simulink model "HvacHeatrTCtrl"  (R2022b)
settings: Solver=FixedStepDiscrete  StartTime=0.0  StopTime=10.0  FixedStep=0.2  RelTol=1e-3
(part 2 of 2)

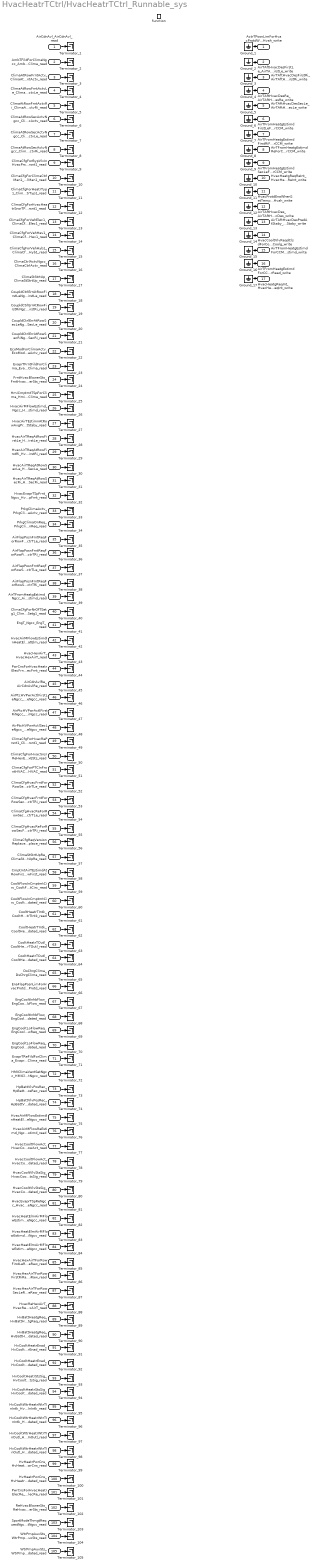
== system HvacHeatrTCtrl/HvacHeatrTCtrl_Runnable_sys ==
  AirCdnAvl_AirCdnAvl_read : Inport
  AmbTFildForClimaNgcc_AmbTFildForClima_read : Inport  Port=2
  ClimaAtRowFrntActv_ClimaAtRowFrntActv_read : Inport  Port=3
  ClimaAtRowFrntActvLe_ClimaAtRowFrntActvLe_read : Inport  Port=4
  ClimaAtRowFrntActvRi_ClimaAtRowFrntActvRi_read : Inport  Port=5
  ClimaAtRowSecActvNgcc_ClimaAtRowSecActv_read : Inport  Port=6
  ClimaAtRowSecActvNgcc_ClimaAtRowSecActvLe_read : Inport  Port=7
  ClimaAtRowSecActvNgcc_ClimaAtRowSecActvRi_read : Inport  Port=8
  ClimaCfgForBypVlvInHvacPrsnt1_ClimaCfgForBypVlvInHvacPrsnt1_read : Inport  Port=9
  ClimaCfgForClimaCtrlMan1_ClimaCfgForClimaCtrlMan1_read : Inport  Port=10
  ClimaCfgForHeatrTyp1_ClimaCfgForHeatrTyp1_read : Inport  Port=11
  ClimaCfgForHvacHeatrSnsrTPrsnt1_ClimaCfgForHvacHeatrSnsrTPrsnt1_read : Inport  Port=12
  ClimaCfgForVehElec1_ClimaCfgForVehElec1_read : Inport  Port=13
  ClimaCfgForVehHev1_ClimaCfgForVehHev1_read : Inport  Port=14
  ClimaCfgForVehHyb1_ClimaCfgForVehHyb1_read : Inport  Port=15
  ClimaCtrlActvNgcc_ClimaCtrlActv_read : Inport  Port=16
  ClimaStStrtUp_ClimaStStrtUp_read : Inport  Port=17
  CoupldCtrlErrAtRowFirstLeNgcc_CoupldCtrlErrAtRowFirstLe_read : Inport  Port=18
  CoupldCtrlErrAtRowFirstRiNgcc_CoupldCtrlErrAtRowFirstRi_read : Inport  Port=19
  CoupldCtrlErrAtRowSecLeNgcc_CoupldCtrlErrAtRowSecLe_read : Inport  Port=20
  CoupldCtrlErrAtRowSecRiNgcc_CoupldCtrlErrAtRowSecRi_read : Inport  Port=21
  EcoModForClimaActv_EcoModForClimaActv_read : Inport  Port=22
  EvaprTFrntFildForClima_EvaprTFrntFildForClima_read : Inport  Port=23
  FrntHvacBlowerSts_FrntHvacBlowerSts_read : Inport  Port=24
  HmiCmptmtTSpForClima_HmiCmptmtTSpForClima_read : Inport  Port=25
  HvacAirMFlowEstimd_Ngcc_HvacAirMFlowEstimd_read : Inport  Port=26
  HvacAirTEstimnAtRowAvgPredAtStaby_HvacAirTEstimnAtRowAvgPredAtStaby_read : Inport  Port=27
  HvacAirTReqAtRowFirstLe_HvacAirTReqAtRowFirstLe_read : Inport  Port=28
  HvacAirTReqAtRowFirstRi_HvacAirTReqAtRowFirstRi_read : Inport  Port=29
  HvacAirTReqAtRowSecLe_HvacAirTReqAtRowSecLe_read : Inport  Port=30
  HvacAirTReqAtRowSecRi_HvacAirTReqAtRowSecRi_read : Inport  Port=31
  HvacEvaprTSpFrnt_Ngcc_HvacEvaprTSpFrnt_read : Inport  Port=32
  PrkgClimaActv_PrkgClimaActv_read : Inport  Port=33
  PrkgClimaOnReq_PrkgClimaOnReq_read : Inport  Port=34
  AirFlapPosnFrntReqForRowFirstForActrTLe_AirFlapPosnFrntReqForRowFirstForActrTLe_read : Inport  Port=35
  AirFlapPosnFrntReqForRowFirstForActrTRi_AirFlapPosnFrntReqForRowFirstForActrTRi_read : Inport  Port=36
  AirFlapPosnFrntReqForRowSecForActrTLe_AirFlapPosnFrntReqForRowSecForActrTLe_read : Inport  Port=37
  AirFlapPosnFrntReqForRowSecForActrTRi_AirFlapPosnFrntReqForRowSecForActrTRi_read : Inport  Port=38
  AirTFromHeatgEstimd_Ngcc_AirTFromHeatgEstimd_read : Inport  Port=39
  ClimaCfgForNrOfTSetg1_ClimaCfgForNrOfTSetg1_read : Inport  Port=40
  EngT_Ngcc_EngT_read : Inport  Port=41
  HvacAirMFlowEstimdInHeatElm_HvacAirMFlowEstimdInHeatElm_read : Inport  Port=42
  HvacHexAirT_HvacHexAirT_read : Inport  Port=43
  PwrCnsForHvacHeatrElecFrnt_PwrCnsForHvacHeatrElecFrnt_read : Inport  Port=44
  AirCdnAvlRe_AirCdnAvlRe_read : Inport  Port=45
  AirPtcHVPwrActlFirstLeNgcc_AirPtcHVPwrActlFirstLeNgcc_read : Inport  Port=46
  AirPtcHVPwrActlFirstRiNgcc_AirPtcHVPwrActlFirstRiNgcc_read : Inport  Port=47
  AirPtcHVPwrActlSecLeNgcc_AirPtcHVPwrActlSecLeNgcc_read : Inport  Port=48
  ClimaCfgForHvacRePrsnt1_ClimaCfgForHvacRePrsnt1_read : Inport  Port=49
  ClimaCfgForHvacSnsrReHexESts_ClimaCfgForHvacSnsrReHexESts_read : Inport  Port=50
  ClimaCfgForPTCInFrontHVAC_ClimaCfgForPTCInFrontHVAC_read : Inport  Port=51
  ClimaCfgHvacFrntForRowSecForActrTLe_ClimaCfgHvacFrntForRowSecForActrTLe_read : Inport  Port=52
  ClimaCfgHvacFrntForRowSecForActrTRi_ClimaCfgHvacFrntForRowSecForActrTRi_read : Inport  Port=53
  ClimaCfgHvacReForRowSecForActrTLe_ClimaCfgHvacReForRowSecForActrTLe_read : Inport  Port=54
  ClimaCfgHvacReForRowSecForActrTRi_ClimaCfgHvacReForRowSecForActrTRi_read : Inport  Port=55
  ClimaCfgReqVersionReplace_ClimaCfgReqVersionReplace_read : Inport  Port=56
  ClimaStStrtUpRe_ClimaStStrtUpRe_read : Inport  Port=57
  CmptmtAirTEstimdAtRowFirst_CmptmtAirTEstimdAtRowFirst_read : Inport  Port=58
  CooltFlowInCmptmtCirc_CooltFlowInCmptmtCirc_read : Inport  Port=59
  CooltFlowInCmptmtCirc_CooltFlowInCmptmtCirc_IsUpdated_read : Inport  Port=60
  CooltHeatrTIntk_CooltHeatrTIntk_read : Inport  Port=61
  CooltHeatrTIntk_CooltHeatrTIntk_IsUpdated_read : Inport  Port=62
  CooltHeatrTOutl_CooltHeatrTOutl_read : Inport  Port=63
  CooltHeatrTOutl_CooltHeatrTOutl_IsUpdated_read : Inport  Port=64
  DsChrgClima_DsChrgClima_read : Inport  Port=65
  EnaFlapPosnLimForHvacProtd_EnaFlapPosnLimForHvacProtd_read : Inport  Port=66
  EngCooltInhbFlow_EngCooltInhbFlow_read : Inport  Port=67
  EngCooltInhbFlow_EngCooltInhbFlow_IsUpdated_read : Inport  Port=68
  EngCooltLoFlowReq_EngCooltLoFlowReq_read : Inport  Port=69
  EngCooltLoFlowReq_EngCooltLoFlowReq_IsUpdated_read : Inport  Port=70
  EvaprTReFildForClima_EvaprTReFildForClima_read : Inport  Port=71
  HMIClimaVentSetNgcc_HMIClimaVentSetNgcc_read : Inport  Port=72
  HpBattVlvPosRec_HpBattVlvPosRec_read : Inport  Port=73
  HpBattVlvPosRec_HpBattVlvPosRec_IsUpdated_read : Inport  Port=74
  HvacAirMFlowEstimdInHeatElmReNgcc_HvacAirMFlowEstimdInHeatElmReNgcc_read : Inport  Port=75
  HvacAirMFlowReEstimd_Ngcc_HvacAirMFlowReEstimd_read : Inport  Port=76
  HvacCooltFlowAct_HvacCooltFlowAct_read : Inport  Port=77
  HvacCooltFlowAct_HvacCooltFlowAct_IsUpdated_read : Inport  Port=78
  HvacCooltVlvStsSig_HvacCooltVlvStsSig_read : Inport  Port=79
  HvacCooltVlvStsSig_HvacCooltVlvStsSig_IsUpdated_read : Inport  Port=80
  HvacEvaprTSpReNgcc_HvacEvaprTSpReNgcc_read : Inport  Port=81
  HvacHeatElmAirMFlowEstimdFirstLeNgcc_HvacHeatElmAirMFlowEstimdFirstLeNgcc_read : Inport  Port=82
  HvacHeatElmAirMFlowEstimdFirstRiNgcc_HvacHeatElmAirMFlowEstimdFirstRiNgcc_read : Inport  Port=83
  HvacHeatElmAirMFlowEstimdSecLeNgcc_HvacHeatElmAirMFlowEstimdSecLeNgcc_read : Inport  Port=84
  HvacHexAirTForRowFirstLeRaw_HvacHexAirTForRowFirstLeRaw_read : Inport  Port=85
  HvacHexAirTForRowFirstRiRaw_HvacHexAirTForRowFirstRiRaw_read : Inport  Port=86
  HvacHexAirTForRowSecLeRaw_HvacHexAirTForRowSecLeRaw_read : Inport  Port=87
  HvacReHexAirT_HvacReHexAirT_read : Inport  Port=88
  HvBattHeatgReq_HvBattHeatgReq_read : Inport  Port=89
  HvBattHeatgReq_HvBattHeatgReq_IsUpdated_read : Inport  Port=90
  HvCooltHeatrEnad_HvCooltHeatrEnad_read : Inport  Port=91
  HvCooltHeatrEnad_HvCooltHeatrEnad_IsUpdated_read : Inport  Port=92
  HvCooltHeatrStsSig_HvCooltHeatrStsSig_read : Inport  Port=93
  HvCooltHeatrStsSig_HvCooltHeatrStsSig_IsUpdated_read : Inport  Port=94
  HvCooltWtrHeatrWtrTInIntk_HvCooltWtrHeatrWtrTInIntk_read : Inport  Port=95
  HvCooltWtrHeatrWtrTInIntk_HvCooltWtrHeatrWtrTInIntk_IsUpdated_read : Inport  Port=96
  HvCooltWtrHeatrWtrTInOutl_HvCooltWtrHeatrWtrTInOutl_read : Inport  Port=97
  HvCooltWtrHeatrWtrTInOutl_HvCooltWtrHeatrWtrTInOutl_IsUpdated_read : Inport  Port=98
  HvHeatrPwrCns_HvHeatrPwrCns_read : Inport  Port=99
  HvHeatrPwrCns_HvHeatrPwrCns_IsUpdated_read : Inport  Port=100
  PwrCnsForHvacHeatrElecRe_PwrCnsForHvacHeatrElecRe_read : Inport  Port=101
  ReHvacBlowerSts_ReHvacBlowerSts_read : Inport  Port=102
  SportModeThmgtRequestNgcc_SportModeThmgtRequestNgcc_read : Inport  Port=103
  WtrPmpAuxSts_WtrPmpAuxSts_read : Inport  Port=104
  WtrPmpAuxSts_WtrPmpAuxSts_IsUpdated_read : Inport  Port=105
  function : TriggerPort  TriggerType=function-call  VariantControl=(inherit)  SampleTimeType=periodic  SampleTime=-1
  Ground_{1..17} (17 alike) : Ground
  Terminator_{1..105} (105 alike) : Terminator
  ActrTPosnLimForHvacProtdWhenSetTempDiffWithHvah_ActrTPosnLimForHvacProtdWhenSetTempDiffWithHvah_write : Outport
      settings: EnsureOutportIsVirtual=on
  AirTAftHvacDesFirstLe_AirTAftHvacDesFirstLe_write : Outport  Port=2
      settings: EnsureOutportIsVirtual=on
  AirTAftHvacDesFirstRi_AirTAftHvacDesFirstRi_write : Outport  Port=3
      settings: EnsureOutportIsVirtual=on
  AirTAftHvacDesRe_AirTAftHvacDesRe_write : Outport  Port=4
      settings: EnsureOutportIsVirtual=on
  AirTAftHvacDesSecLe_AirTAftHvacDesSecLe_write : Outport  Port=5
      settings: EnsureOutportIsVirtual=on
  AirTFromHeatgEstimdFirstLeForCCM_AirTFromHeatgEstimdFirstLeForCCM_write : Outport  Port=6
      settings: EnsureOutportIsVirtual=on
  AirTFromHeatgEstimdFirstRiForCCM_AirTFromHeatgEstimdFirstRiForCCM_write : Outport  Port=7
      settings: EnsureOutportIsVirtual=on
  AirTFromHeatgEstimdReForCCM_AirTFromHeatgEstimdReForCCM_write : Outport  Port=8
      settings: EnsureOutportIsVirtual=on
  AirTFromHeatgEstimdSecLeForCCM_AirTFromHeatgEstimdSecLeForCCM_write : Outport  Port=9
      settings: EnsureOutportIsVirtual=on
  HvacHeatgReqReInt_HvacHeatgReqReInt_write : Outport  Port=10
      settings: EnsureOutportIsVirtual=on
  HvacProtdEnaWhenSetTempDiffWithHvah_HvacProtdEnaWhenSetTempDiffWithHvah_write : Outport  Port=11
      settings: EnsureOutportIsVirtual=on
  AirTAftHvacDes_AirTAftHvacDes_write : Outport  Port=12
      settings: EnsureOutportIsVirtual=on
  AirTAftHvacDesPredAtStaby_AirTAftHvacDesPredAtStaby_write : Outport  Port=13
      settings: EnsureOutportIsVirtual=on
  HvacCooltVlvReqdClsdForCoolg_HvacCooltVlvReqdClsdForCoolg_write : Outport  Port=14
      settings: EnsureOutportIsVirtual=on
  AirTFromHeatgEstimdForCCM_AirTFromHeatgEstimd_write : Outport  Port=15
      settings: EnsureOutportIsVirtual=on
  AirTFromHeatgEstimdForCCMForRead_AirTFromHeatgEstimdForCCMForRead_write : Outport  Port=16
      settings: EnsureOutportIsVirtual=on
  HvacHeatgReqInt_HvacHeatgReqInt_write : Outport  Port=17
      settings: EnsureOutportIsVirtual=on
  wires:
  AirCdnAvl_AirCdnAvl_read/1 -> Terminator_1/1
  AmbTFildForClimaNgcc_AmbTFildForClima_read/1 -> Terminator_2/1
  ClimaAtRowFrntActv_ClimaAtRowFrntActv_read/1 -> Terminator_3/1
  ClimaAtRowFrntActvLe_ClimaAtRowFrntActvLe_read/1 -> Terminator_4/1
  ClimaAtRowFrntActvRi_ClimaAtRowFrntActvRi_read/1 -> Terminator_5/1
  ClimaAtRowSecActvNgcc_ClimaAtRowSecActv_read/1 -> Terminator_6/1
  ClimaAtRowSecActvNgcc_ClimaAtRowSecActvLe_read/1 -> Terminator_7/1
  ClimaAtRowSecActvNgcc_ClimaAtRowSecActvRi_read/1 -> Terminator_8/1
  ClimaCfgForBypVlvInHvacPrsnt1_ClimaCfgForBypVlvInHvacPrsnt1_read/1 -> Terminator_9/1
  ClimaCfgForClimaCtrlMan1_ClimaCfgForClimaCtrlMan1_read/1 -> Terminator_10/1
  ClimaCfgForHeatrTyp1_ClimaCfgForHeatrTyp1_read/1 -> Terminator_11/1
  ClimaCfgForHvacHeatrSnsrTPrsnt1_ClimaCfgForHvacHeatrSnsrTPrsnt1_read/1 -> Terminator_12/1
  ClimaCfgForVehElec1_ClimaCfgForVehElec1_read/1 -> Terminator_13/1
  ClimaCfgForVehHev1_ClimaCfgForVehHev1_read/1 -> Terminator_14/1
  ClimaCfgForVehHyb1_ClimaCfgForVehHyb1_read/1 -> Terminator_15/1
  ClimaCtrlActvNgcc_ClimaCtrlActv_read/1 -> Terminator_16/1
  ClimaStStrtUp_ClimaStStrtUp_read/1 -> Terminator_17/1
  CoupldCtrlErrAtRowFirstLeNgcc_CoupldCtrlErrAtRowFirstLe_read/1 -> Terminator_18/1
  CoupldCtrlErrAtRowFirstRiNgcc_CoupldCtrlErrAtRowFirstRi_read/1 -> Terminator_19/1
  CoupldCtrlErrAtRowSecLeNgcc_CoupldCtrlErrAtRowSecLe_read/1 -> Terminator_20/1
  CoupldCtrlErrAtRowSecRiNgcc_CoupldCtrlErrAtRowSecRi_read/1 -> Terminator_21/1
  EcoModForClimaActv_EcoModForClimaActv_read/1 -> Terminator_22/1
  EvaprTFrntFildForClima_EvaprTFrntFildForClima_read/1 -> Terminator_23/1
  FrntHvacBlowerSts_FrntHvacBlowerSts_read/1 -> Terminator_24/1
  HmiCmptmtTSpForClima_HmiCmptmtTSpForClima_read/1 -> Terminator_25/1
  HvacAirMFlowEstimd_Ngcc_HvacAirMFlowEstimd_read/1 -> Terminator_26/1
  HvacAirTEstimnAtRowAvgPredAtStaby_HvacAirTEstimnAtRowAvgPredAtStaby_read/1 -> Terminator_27/1
  HvacAirTReqAtRowFirstLe_HvacAirTReqAtRowFirstLe_read/1 -> Terminator_28/1
  HvacAirTReqAtRowFirstRi_HvacAirTReqAtRowFirstRi_read/1 -> Terminator_29/1
  HvacAirTReqAtRowSecLe_HvacAirTReqAtRowSecLe_read/1 -> Terminator_30/1
  HvacAirTReqAtRowSecRi_HvacAirTReqAtRowSecRi_read/1 -> Terminator_31/1
  HvacEvaprTSpFrnt_Ngcc_HvacEvaprTSpFrnt_read/1 -> Terminator_32/1
  PrkgClimaActv_PrkgClimaActv_read/1 -> Terminator_33/1
  PrkgClimaOnReq_PrkgClimaOnReq_read/1 -> Terminator_34/1
  AirFlapPosnFrntReqForRowFirstForActrTLe_AirFlapPosnFrntReqForRowFirstForActrTLe_read/1 -> Terminator_35/1
  AirFlapPosnFrntReqForRowFirstForActrTRi_AirFlapPosnFrntReqForRowFirstForActrTRi_read/1 -> Terminator_36/1
  AirFlapPosnFrntReqForRowSecForActrTLe_AirFlapPosnFrntReqForRowSecForActrTLe_read/1 -> Terminator_37/1
  AirFlapPosnFrntReqForRowSecForActrTRi_AirFlapPosnFrntReqForRowSecForActrTRi_read/1 -> Terminator_38/1
  AirTFromHeatgEstimd_Ngcc_AirTFromHeatgEstimd_read/1 -> Terminator_39/1
  ClimaCfgForNrOfTSetg1_ClimaCfgForNrOfTSetg1_read/1 -> Terminator_40/1
  EngT_Ngcc_EngT_read/1 -> Terminator_41/1
  HvacAirMFlowEstimdInHeatElm_HvacAirMFlowEstimdInHeatElm_read/1 -> Terminator_42/1
  HvacHexAirT_HvacHexAirT_read/1 -> Terminator_43/1
  PwrCnsForHvacHeatrElecFrnt_PwrCnsForHvacHeatrElecFrnt_read/1 -> Terminator_44/1
  AirCdnAvlRe_AirCdnAvlRe_read/1 -> Terminator_45/1
  AirPtcHVPwrActlFirstLeNgcc_AirPtcHVPwrActlFirstLeNgcc_read/1 -> Terminator_46/1
  AirPtcHVPwrActlFirstRiNgcc_AirPtcHVPwrActlFirstRiNgcc_read/1 -> Terminator_47/1
  AirPtcHVPwrActlSecLeNgcc_AirPtcHVPwrActlSecLeNgcc_read/1 -> Terminator_48/1
  ClimaCfgForHvacRePrsnt1_ClimaCfgForHvacRePrsnt1_read/1 -> Terminator_49/1
  ClimaCfgForHvacSnsrReHexESts_ClimaCfgForHvacSnsrReHexESts_read/1 -> Terminator_50/1
  ClimaCfgForPTCInFrontHVAC_ClimaCfgForPTCInFrontHVAC_read/1 -> Terminator_51/1
  ClimaCfgHvacFrntForRowSecForActrTLe_ClimaCfgHvacFrntForRowSecForActrTLe_read/1 -> Terminator_52/1
  ClimaCfgHvacFrntForRowSecForActrTRi_ClimaCfgHvacFrntForRowSecForActrTRi_read/1 -> Terminator_53/1
  ClimaCfgHvacReForRowSecForActrTLe_ClimaCfgHvacReForRowSecForActrTLe_read/1 -> Terminator_54/1
  ClimaCfgHvacReForRowSecForActrTRi_ClimaCfgHvacReForRowSecForActrTRi_read/1 -> Terminator_55/1
  ClimaCfgReqVersionReplace_ClimaCfgReqVersionReplace_read/1 -> Terminator_56/1
  ClimaStStrtUpRe_ClimaStStrtUpRe_read/1 -> Terminator_57/1
  CmptmtAirTEstimdAtRowFirst_CmptmtAirTEstimdAtRowFirst_read/1 -> Terminator_58/1
  CooltFlowInCmptmtCirc_CooltFlowInCmptmtCirc_read/1 -> Terminator_59/1
  CooltFlowInCmptmtCirc_CooltFlowInCmptmtCirc_IsUpdated_read/1 -> Terminator_60/1
  CooltHeatrTIntk_CooltHeatrTIntk_read/1 -> Terminator_61/1
  CooltHeatrTIntk_CooltHeatrTIntk_IsUpdated_read/1 -> Terminator_62/1
  CooltHeatrTOutl_CooltHeatrTOutl_read/1 -> Terminator_63/1
  CooltHeatrTOutl_CooltHeatrTOutl_IsUpdated_read/1 -> Terminator_64/1
  DsChrgClima_DsChrgClima_read/1 -> Terminator_65/1
  EnaFlapPosnLimForHvacProtd_EnaFlapPosnLimForHvacProtd_read/1 -> Terminator_66/1
  EngCooltInhbFlow_EngCooltInhbFlow_read/1 -> Terminator_67/1
  EngCooltInhbFlow_EngCooltInhbFlow_IsUpdated_read/1 -> Terminator_68/1
  EngCooltLoFlowReq_EngCooltLoFlowReq_read/1 -> Terminator_69/1
  EngCooltLoFlowReq_EngCooltLoFlowReq_IsUpdated_read/1 -> Terminator_70/1
  EvaprTReFildForClima_EvaprTReFildForClima_read/1 -> Terminator_71/1
  HMIClimaVentSetNgcc_HMIClimaVentSetNgcc_read/1 -> Terminator_72/1
  HpBattVlvPosRec_HpBattVlvPosRec_read/1 -> Terminator_73/1
  HpBattVlvPosRec_HpBattVlvPosRec_IsUpdated_read/1 -> Terminator_74/1
  HvacAirMFlowEstimdInHeatElmReNgcc_HvacAirMFlowEstimdInHeatElmReNgcc_read/1 -> Terminator_75/1
  HvacAirMFlowReEstimd_Ngcc_HvacAirMFlowReEstimd_read/1 -> Terminator_76/1
  HvacCooltFlowAct_HvacCooltFlowAct_read/1 -> Terminator_77/1
  HvacCooltFlowAct_HvacCooltFlowAct_IsUpdated_read/1 -> Terminator_78/1
  HvacCooltVlvStsSig_HvacCooltVlvStsSig_read/1 -> Terminator_79/1
  HvacCooltVlvStsSig_HvacCooltVlvStsSig_IsUpdated_read/1 -> Terminator_80/1
  HvacEvaprTSpReNgcc_HvacEvaprTSpReNgcc_read/1 -> Terminator_81/1
  HvacHeatElmAirMFlowEstimdFirstLeNgcc_HvacHeatElmAirMFlowEstimdFirstLeNgcc_read/1 -> Terminator_82/1
  HvacHeatElmAirMFlowEstimdFirstRiNgcc_HvacHeatElmAirMFlowEstimdFirstRiNgcc_read/1 -> Terminator_83/1
  HvacHeatElmAirMFlowEstimdSecLeNgcc_HvacHeatElmAirMFlowEstimdSecLeNgcc_read/1 -> Terminator_84/1
  HvacHexAirTForRowFirstLeRaw_HvacHexAirTForRowFirstLeRaw_read/1 -> Terminator_85/1
  HvacHexAirTForRowFirstRiRaw_HvacHexAirTForRowFirstRiRaw_read/1 -> Terminator_86/1
  HvacHexAirTForRowSecLeRaw_HvacHexAirTForRowSecLeRaw_read/1 -> Terminator_87/1
  HvacReHexAirT_HvacReHexAirT_read/1 -> Terminator_88/1
  HvBattHeatgReq_HvBattHeatgReq_read/1 -> Terminator_89/1
  HvBattHeatgReq_HvBattHeatgReq_IsUpdated_read/1 -> Terminator_90/1
  HvCooltHeatrEnad_HvCooltHeatrEnad_read/1 -> Terminator_91/1
  HvCooltHeatrEnad_HvCooltHeatrEnad_IsUpdated_read/1 -> Terminator_92/1
  HvCooltHeatrStsSig_HvCooltHeatrStsSig_read/1 -> Terminator_93/1
  HvCooltHeatrStsSig_HvCooltHeatrStsSig_IsUpdated_read/1 -> Terminator_94/1
  HvCooltWtrHeatrWtrTInIntk_HvCooltWtrHeatrWtrTInIntk_read/1 -> Terminator_95/1
  HvCooltWtrHeatrWtrTInIntk_HvCooltWtrHeatrWtrTInIntk_IsUpdated_read/1 -> Terminator_96/1
  HvCooltWtrHeatrWtrTInOutl_HvCooltWtrHeatrWtrTInOutl_read/1 -> Terminator_97/1
  HvCooltWtrHeatrWtrTInOutl_HvCooltWtrHeatrWtrTInOutl_IsUpdated_read/1 -> Terminator_98/1
  HvHeatrPwrCns_HvHeatrPwrCns_read/1 -> Terminator_99/1
  HvHeatrPwrCns_HvHeatrPwrCns_IsUpdated_read/1 -> Terminator_100/1
  PwrCnsForHvacHeatrElecRe_PwrCnsForHvacHeatrElecRe_read/1 -> Terminator_101/1
  ReHvacBlowerSts_ReHvacBlowerSts_read/1 -> Terminator_102/1
  SportModeThmgtRequestNgcc_SportModeThmgtRequestNgcc_read/1 -> Terminator_103/1
  WtrPmpAuxSts_WtrPmpAuxSts_read/1 -> Terminator_104/1
  WtrPmpAuxSts_WtrPmpAuxSts_IsUpdated_read/1 -> Terminator_105/1
  Ground_1/1 -> ActrTPosnLimForHvacProtdWhenSetTempDiffWithHvah_ActrTPosnLimForHvacProtdWhenSetTempDiffWithHvah_write/1
  Ground_2/1 -> AirTAftHvacDesFirstLe_AirTAftHvacDesFirstLe_write/1
  Ground_3/1 -> AirTAftHvacDesFirstRi_AirTAftHvacDesFirstRi_write/1
  Ground_4/1 -> AirTAftHvacDesRe_AirTAftHvacDesRe_write/1
  Ground_5/1 -> AirTAftHvacDesSecLe_AirTAftHvacDesSecLe_write/1
  Ground_6/1 -> AirTFromHeatgEstimdFirstLeForCCM_AirTFromHeatgEstimdFirstLeForCCM_write/1
  Ground_7/1 -> AirTFromHeatgEstimdFirstRiForCCM_AirTFromHeatgEstimdFirstRiForCCM_write/1
  Ground_8/1 -> AirTFromHeatgEstimdReForCCM_AirTFromHeatgEstimdReForCCM_write/1
  Ground_9/1 -> AirTFromHeatgEstimdSecLeForCCM_AirTFromHeatgEstimdSecLeForCCM_write/1
  Ground_10/1 -> HvacHeatgReqReInt_HvacHeatgReqReInt_write/1
  Ground_11/1 -> HvacProtdEnaWhenSetTempDiffWithHvah_HvacProtdEnaWhenSetTempDiffWithHvah_write/1
  Ground_12/1 -> AirTAftHvacDes_AirTAftHvacDes_write/1
  Ground_13/1 -> AirTAftHvacDesPredAtStaby_AirTAftHvacDesPredAtStaby_write/1
  Ground_14/1 -> HvacCooltVlvReqdClsdForCoolg_HvacCooltVlvReqdClsdForCoolg_write/1
  Ground_15/1 -> AirTFromHeatgEstimdForCCM_AirTFromHeatgEstimd_write/1
  Ground_16/1 -> AirTFromHeatgEstimdForCCMForRead_AirTFromHeatgEstimdForCCMForRead_write/1
  Ground_17/1 -> HvacHeatgReqInt_HvacHeatgReqInt_write/1
